simulink model "FlightSim"  (R2023a)
settings: Solver=VariableStepAuto  StartTime=0.0  StopTime=20  RelTol=1e-3
model workspace (MAT data in the model file):
  polyCoefficient = [1 1 1]
values from neighbouring files (symbols this model uses):
  airDensity = 1.2250    (= 1.225)    [FlightSimSetup.m]
  airKinematicViscosity = 1.48*10^-5    (= 1.48e-05)    [FlightSimSetup.m]
  AOA = deg2rad(0)    [FlightSimSetup.m]
  axialDistanceMACCGx = abs(finMACLeadingEdgeLocation) + finLeadingEdgeCGxlength    [finParameters.m]
  bodyDiameter = 2/100    (= 0.02)    [FlightSimSetup.m]
  bodyLength = 20/100    (= 0.2)    [FlightSimSetup.m]
  bodyLongitudinalInertia = bodyMass*( ((bodyDiameter/2).^2)/4 + ((bodyLength).^2)/12)    [InertiaParameters.m]
  bodyMass = 12/1000    (= 0.012)    [FlightSimSetup.m]
  bodyPlanformArea = bodyDiameter*bodyLength    (= 0.004)    [FlightSimSetup.m]
  bodyRoughness = 60*10^-6    (= 6e-05)    [FlightSimSetup.m]
  casingLongitudinalInertia = motorCasingMass/12*(3*(motorOuterRadius.^2+motorInnerRadius.^2)+motorLength.^2)    [InertiaParameters.m]
  cYaw = 0    [FlightSimSetup.m]
  dIdt = zeros(3)    [InertiaParameters.m]
  finArea = (finRootChord+finTailChord)*finSpan/2    [finParameters.m]
  finCantAngle = deg2rad(0)    [FlightSimSetup.m]
  finCentroidAxial = finSpan/3 * ( (finRootChord + 2*finTailChord)/(finRootChord + finTailChord) ) - backOffset    [finParameters.m]
  finCount = 4    [FlightSimSetup.m]
  finFrontSweepAngle = acos(dot(frontSweepAngleRefVector1, frontSweepAngleRefVector2) / (norm(frontSweepAngleRefVector1) * norm(frontSweepAngleRefVector2)))    [finParameters.m]
  finLeadingEdgeNoseConeDistance = bodyLength + noseConeHeight - finRootChord + backOffset    [finParameters.m]
  finLongitudinalInertia = finLongitudinalInertia * singleFinMass * finCount    [InertiaParameters.m]
  finMACLeadingEdgeLocation = abs( integral(finMACLeadingEdgeLocationFunction, 0, finSpan)/finArea )    [finParameters.m]
  finMACLength = integral(chordLengthFunction, 0, finSpan)/finArea    [finParameters.m]
  finMACNoseconeAxialDistance = finLeadingEdgeNoseConeDistance + finMACLeadingEdgeLocation+ 1/2*finMACLength    [finParameters.m]
  finMidChordSweepAngle = acos(dot(midChordAngleRefVector1, midChordAngleRefVector2) / (norm(midChordAngleRefVector1) * norm(midChordAngleRefVector2)))    [finParameters.m]
  finProfileType = 3    [FlightSimSetup.m]
  finRootChord = abs(P_frontRoot(2)-P_backRoot(2))    [finParameters.m]
  finSpan = abs(P_frontTrail(1)- P_frontRoot(1))    [finParameters.m]
  finTailChord = abs(P_frontTrail(2)-P_backTrail(2))    [finParameters.m]
  finThickness = 0.15/100    (= 0.0015)    [FlightSimSetup.m]
  finX_t = P_frontRoot(2) - P_frontTrail(2)    [finParameters.m]
  launchLugInnerDiameter = 0    [FlightSimSetup.m]
  launchLugLength = 0    [FlightSimSetup.m]
  launchLugOuterDiameter = 0    [FlightSimSetup.m]
  machNum = 0    [FlightSimSetup.m]
  motorCasingMass = motorInitialTotalMass - motorInitialPropMass    (= 0.071)    [FlightSimSetup.m]
  motorInitialPropMass = 83/1000    (= 0.083)    [FlightSimSetup.m]
  motorInnerRadius = motorOuterRadius - casingThickness    [InertiaParameters.m]
  motorLength = 156/1000    (= 0.156)    [FlightSimSetup.m]
  motorTotalImpulse = 129.9    [FlightSimSetup.m]
  noseConeHeight = 6/100    (= 0.06)    [FlightSimSetup.m]
  noseConeJoinAngle = pi/2 - atan(noseConeHeight/(bodyDiameter/2))    [FlightSimSetup.m]
  noseConeLongitudinalInertia = noseConeLongitudinalInertia*noseConeMass    [noseConeParameters.m]
  noseConeMass = 5/1000    (= 0.005)    [FlightSimSetup.m]
  noseConePlanformArea = (1/2)*bodyDiameter* noseConeHeight    [noseConeParameters.m]
  noseConeType = 8    [FlightSimSetup.m]
  noseConeVolume = (1/3)*noseConeHeight*(bodyDiameter/2)^2*pi    [noseConeParameters.m]
  noseConeWettedArea = pi*sqrt( (bodyDiameter/2)^2 + noseConeHeight^2)*bodyDiameter/2    [noseConeParameters.m]
  pitchCenterX = 0    [FlightSimSetup.m]
  pitchRate = 0    [FlightSimSetup.m]
  radialMACPosition = finMACSpanwisePosition + bodyDiameter/2    [finParameters.m]
  refArea = bodyDiameter^2*pi/4    (= 0.000314159265359)    [FlightSimSetup.m]
  rocketLength = (20+6)/100    (= 0.26)    [FlightSimSetup.m]
  rocketNonMotorMass = noseConeMass + bodyMass + totalFinMass    (= 0.021)    [FlightSimSetup.m]
  rocketRotationalInertia = bodyRotationalInertia + casingRotationalInertia + finRotationalInertia + noseConeLongitudinalInertia    [InertiaParameters.m]
  rocketVelocityMag = machNum*340.17    (= 0)    [FlightSimSetup.m]
  rollRate = 0    [FlightSimSetup.m]
  totalFinMass = singleFinMass * finCount    (= 0.004)    [FlightSimSetup.m]
  windAngle = 0    [FlightSimSetup.m]
  yawRate = 0    [FlightSimSetup.m]
libraries: shared6dof x1, aerolibanim x1, aerolibadyn x1, aerolibbdyn x1, sharedutil x1
(part 3 of 3)

== system FlightSim/Aerodyanmic Forces/Normal Force and Pitch Moment Calc/Normal, Cp,Pitch of Nosecone/noseConeLiftFactor ==
  note: assumes nose cone base diameter is same as body diameter (true with no botail)
  note: note: this is slightly different than the equation (notably the sin%2 part). That is because implementation in OPen rocket is different, as shown below from SymmetricComponent.java line 180
  note: Equations
  note: Origin: equ 3.26  of Open rocket Document
      |
      | Description: CNa due to Body Lift, K is a constant roughly
      | equal to 1.1
  note: makes sure that there is no divide by 0 error when AOA = 0,
      | and using small angle approximation when AOA = 0, sin(AOA)/AOA = 1
  AOA : Inport
  noseConePlanformArea : Inport  Port=2
  bodyDiameter : Inport  Port=3
  Aref : Product  Inputs=3
  Constant : Constant  Value=.25
  Constant3 : Constant  Value=0
  Display{,1..3} (4 alike) : Display
  Divide : Product  Inputs=*/
  Divide3 : Product  Inputs=*/
  K : Constant  Value=1.1
  Lift : Product  Inputs=3
  Pi : Constant  Value=pi  OutDataTypeStr=Inherit: Inherit via back propagation
  Sin1 : Trigonometry
  Square : Math  Operator=square
  Square1 : Math  Operator=square
  Switch1 : Switch  Criteria=u2 > Threshold  Threshold=0.001  SaturateOnIntegerOverflow=off  InputSameDT=off
  noseConeLift : Outport
  wires:
  noseConePlanformArea/1 -> Display1/1, Divide/1
  bodyDiameter/1 -> Square/1
  Constant/1 -> Aref/3
  Pi/1 -> Aref/2
  Square/1 -> Aref/1
  Aref/1 -> Display2/1, Divide/2
  Divide/1 -> Display/1, Lift/1
  K/1 -> Lift/2
  Lift/1 -> Display3/1, noseConeLift/1
  Switch1/1 -> Lift/3
  Divide3/1 -> Switch1/1
  Constant3/1 -> Switch1/3
  Sin1/1 -> Square1/1
  AOA/1 -> Sin1/1, Switch1/2, Divide3/2
  Square1/1 -> Divide3/1

== system FlightSim/Aerodyanmic Forces/Axial Drag/Body Pressure Drag/FinCD/AirfoilShape ==
  note: only works for mach num < 1
  note: rounded Leading edge Cd
  frontSweepAngle : Inport
  machNum : Inport  Port=2
  Action Port : ActionPort  ActionPortLabel=case [ 2 ]:
  Constant : Constant
  Constant1 : Constant  Value=-.417
  Constant2 : Constant
  Constant3 : Constant  Value=.9
  Constant4 : Constant
  Cos : Trigonometry  Operator=cos
  Gain1 : Gain  Gain=1.785
  Power : Math  Operator=pow
  Product : Product
  Square : Math  Operator=square
  Square1 : Math  Operator=square
  Subtract{,1..3} (4 alike) : Sum  Inputs=+-
  Switch : Switch  Criteria=u2 > Threshold  Threshold=.9  SaturateOnIntegerOverflow=off  InputSameDT=off
  airfoilFinPressureCD : Outport
  wires:
  Constant2/1 -> Subtract1/2
  Subtract/1 -> Power/1
  Constant/1 -> Subtract/1
  Power/1 -> Subtract1/1
  Square/1 -> Subtract/2
  Constant1/1 -> Power/2
  machNum/1 -> Square/1, Subtract2/1, Switch/2
  Constant3/1 -> Subtract2/2
  Subtract2/1 -> Gain1/1
  Gain1/1 -> Subtract3/2
  Constant4/1 -> Subtract3/1
  Subtract3/1 -> Switch/1
  Subtract1/1 -> Switch/3
  Switch/1 -> Product/2
  Product/1 -> airfoilFinPressureCD/1
  frontSweepAngle/1 -> Cos/1
  Cos/1 -> Square1/1
  Square1/1 -> Product/1

== system FlightSim/Aerodyanmic Forces/Axial Drag/Body Pressure Drag/FinCD/RoundShape ==
  note: rounded Leading edge Cd
  note: only works for mach num < 1
  baseDrag : Inport
  frontSweepAngle : Inport  Port=2
  machNum : Inport  Port=3
  Action Port : ActionPort  ActionPortLabel=case [ 1 ]:
  Add : Sum
  Constant : Constant
  Constant1 : Constant  Value=-.417
  Constant2 : Constant
  Constant3 : Constant  Value=.9
  Constant4 : Constant
  Cos : Trigonometry  Operator=cos
  Gain : Gain  Gain=.5
  Gain1 : Gain  Gain=1.785
  Power : Math  Operator=pow
  Product : Product
  Square : Math  Operator=square
  Square1 : Math  Operator=square
  Subtract{,1..3} (4 alike) : Sum  Inputs=+-
  Switch : Switch  Criteria=u2 > Threshold  Threshold=.9  SaturateOnIntegerOverflow=off  InputSameDT=off
  roundFinPressureCD : Outport
  wires:
  Constant2/1 -> Subtract1/2
  Constant1/1 -> Power/2
  machNum/1 -> Square/1, Subtract2/1, Switch/2
  Square/1 -> Subtract/2
  Subtract3/1 -> Switch/1
  Switch/1 -> Product/2
  Constant/1 -> Subtract/1
  Power/1 -> Subtract1/1
  Subtract/1 -> Power/1
  Constant3/1 -> Subtract2/2
  Subtract2/1 -> Gain1/1
  Constant4/1 -> Subtract3/1
  Gain1/1 -> Subtract3/2
  Subtract1/1 -> Switch/3
  baseDrag/1 -> Gain/1
  Gain/1 -> Add/1
  Product/1 -> Add/2
  Add/1 -> roundFinPressureCD/1
  frontSweepAngle/1 -> Cos/1
  Cos/1 -> Square1/1
  Square1/1 -> Product/1

== system FlightSim/Aerodyanmic Forces/Axial Drag/Body Pressure Drag/FinCD/SquareShape ==
  frontSweepAngle : Inport
  stagnationPressure : Inport  Port=2
  baseDrag : Inport  Port=3
  Action Port : ActionPort  ActionPortLabel=case [ 3 ]:
  Add : Sum
  Cos : Trigonometry  Operator=cos
  Display : Display
  Product : Product
  Square : Math  Operator=square
  squareFinPressureCD : Outport
  wires:
  stagnationPressure/1 -> Product/1
  frontSweepAngle/1 -> Cos/1
  Product/1 -> Add/1
  baseDrag/1 -> Add/2
  Add/1 -> Display/1, squareFinPressureCD/1
  Cos/1 -> Square/1
  Square/1 -> Product/2
